simulink model "wlan_phy_rx_pmd"  (Simulink 7.8)
settings: Solver=VariableStepDiscrete  SolverMode=SingleTasking  StartTime=0.0  StopTime=simtime  RelTol=1e-3
PostLoadFcn:
  | wlan_phy_rx_init
InitFcn:
  | wlan_phy_rx_init
StartFcn:
  | wlan_phy_rx_init
values from neighbouring files (symbols this model uses):
  barker_seq20 = 0.95 * circshift(barker_seq20, [0 4]) ./ max(abs(barker_seq20))    [wlan_phy_rx_init.m]
  bit_scrambler_lfsr_bytes = bi2de(reshape(repmat(scr, 1, 8), 8, 127)', 'left-msb')    [wlan_phy_rx_init.m]
  deinterleave_ROM = [deinterleave_ROM interleave_64QAM zeros(1, 512-length(interleave_64QAM))]    [wlan_phy_rx_init.m]
  longCorr_coef_i = [3*real(LTS_corr)]    [wlan_phy_rx_init.m]
  longCorr_coef_q = [3*imag(LTS_corr)]    [wlan_phy_rx_init.m]
  MAX_CP_LEN = 32    [wlan_phy_rx_init.m]
  MAX_NCBPS = 288    [wlan_phy_rx_init.m]
  MAX_NUM_BYTES = 4096    [wlan_phy_rx_init.m]
  MAX_NUM_SC = 64    [wlan_phy_rx_init.m]
  MAX_NUM_SYMS = 600    [wlan_phy_rx_init.m]
  n_bits_preFFT_sampBuff = ceil(log2(4*MAX_NUM_SC))    [wlan_phy_rx_init.m]
  NCBPS_16QAM = 192    [wlan_phy_rx_init.m]
  NCBPS_64QAM = 288    [wlan_phy_rx_init.m]
  NCBPS_BPSK = 48    [wlan_phy_rx_init.m]
  NCBPS_QPSK = 96    [wlan_phy_rx_init.m]
  NDBPS_16QAM12 = 96    [wlan_phy_rx_init.m]
  NDBPS_16QAM34 = 144    [wlan_phy_rx_init.m]
  NDBPS_64QAM23 = 192    [wlan_phy_rx_init.m]
  NDBPS_64QAM34 = 216    [wlan_phy_rx_init.m]
  NDBPS_BPSK12 = 24    [wlan_phy_rx_init.m]
  NDBPS_BPSK34 = 36    [wlan_phy_rx_init.m]
  NDBPS_QPSK12 = 48    [wlan_phy_rx_init.m]
  NDBPS_QPSK34 = 72    [wlan_phy_rx_init.m]
  paylod_vec_samp_time = 8    [wlan_phy_rx_init.m]
  PHY_MIN_PKT_LEN = 14    [wlan_phy_rx_init.m]
  scr_ind_rev = zeros(1,128)    [wlan_phy_rx_init.m]
undefined symbols (not defined in the file or in its folder): gcbh, xlBlockLoadCallback, xlGetNormalizedPeriod, xlipgetpartsetting
libraries: xbsIndex_r4 x3519, simulink x1, xbsEDKLib_r4 x1
(part 1 of 21)
settings Model: Creator=murphpo  LastModifiedBy=murphpo
settings OptimizationCC: InlineParams=on
settings DebuggingCC: UnconnectedOutputMsg=none

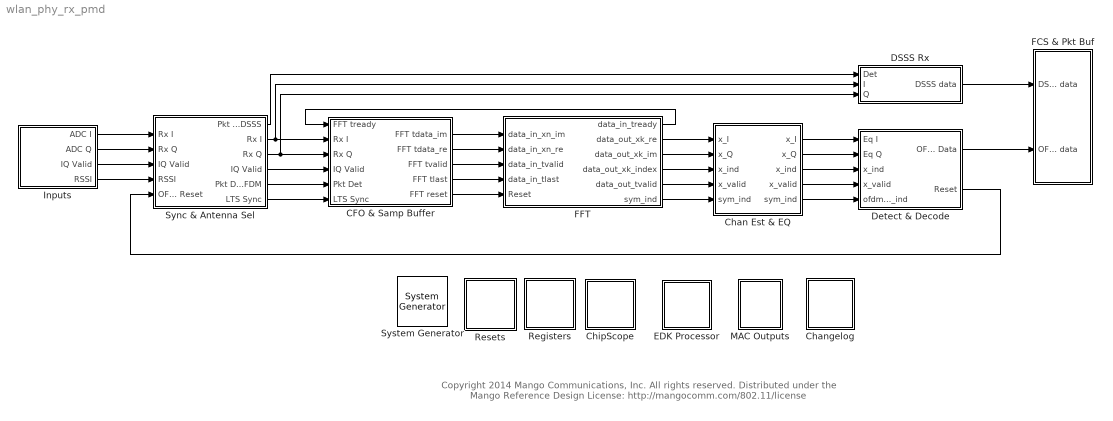
== system wlan_phy_rx_pmd ==
  note: Copyright 2014 Mango Communications, Inc. All rights reserved.
      |
      | Distributed under the Mango Reference Design License:
      | http://mangocomm.com/802.11/license
  " System Generator" : xbsIndex_r4/ System Generator  Tag=genX  xilinxfamily=virtex6  part=xc6vlx240t  speed=-2  package=ff1156  synthesis_tool=XST  clock_wrapper=Clock Enables  directory=./pcore_rx_v282  proj_type=Project Navigator  Synth_file=XST Defaults  Impl_file=ISE Defaults  testbench=off  simulink_period=1  sysclk_period=6.25  dcm_input_clock_period=10  incr_netlist=off  trim_vbits=Everywhere in SubSystem  dbl_ovrd=According to Block Masks  core_generation=According to Block Masks  run_coregen=off  deprecated_control=off  eval_field=0  block_type=sysgen
  CFO & Samp Buffer : Subsystem
  Chan Est & EQ : Subsystem
  Changelog : Subsystem
  ChipScope : Subsystem
  DSSS Rx : Subsystem
  Detect & Decode : Subsystem
  EDK Processor : Subsystem  LoadFcn=xlBlockLoadCallback(gcbh);  mask(Xilinx EDK Processor Block){mode=EDK pcore generation, xmp=, MemVisToProc=<qt bgcolor="#FFFFFF"><div><img src="S:/Xilinx/14.4/ISE_DS/ISE/sysgen/data/images/registerplus.gif"> &lt;&lt;DEBUG_GPIO&gt;&gt;<br></div><div><img src="S:/Xilinx/14.4/ISE_DS/ISE/sysgen/data/images/registerplus.gif"> &lt;&lt;RSSI_THRESH&gt;&gt;<br></div><div><img src="S:/Xilinx/14.4/ISE_DS/ISE/sysgen/data/images/registerplus.gif"> &lt;&lt;CONFIG&gt;&gt;<br></div><div><img src="S:/Xilinx/14.4/ISE_DS/ISE/sysgen/data/images/registerplus.gif"> &lt;&lt;DSSS_RX_CONFIG&gt;&gt;<br></div><div><img src="S:/Xilinx/14.4/ISE_DS/ISE/sysgen/data/images/registerplus.gif"> &lt;&lt;PKT_BUF_SEL&gt;&gt;<br></div><div><img src="S:/Xilinx/14.4/ISE_DS/ISE/sysgen/data/images/registerplus.gif"> &lt;&lt;PKTDET_AUTOCORR_CONFIG&gt;&gt;<br></div><div><img src="S:/Xilinx/14.4/ISE_DS/ISE/sysgen/data/images/registerplus.gif"> &lt;&lt;FEC_CONFIG&gt;&gt;<br></div><div><img src="S:/Xilinx/14.4/ISE_DS/ISE/sysgen/data/images/registerplus.gif"> &lt;&lt;Control&gt;&gt;<br></div><div><img src="S:/Xilinx/14.4/ISE_DS/ISE/sysgen/data/images/registerplus.gif"> &lt;&lt;LTS_Corr_Thresh&gt;&gt;<br></div><div><img src="S:/Xilinx/14.4/ISE_DS/ISE/sysgen/data/images/registerplus.gif"> &lt;&lt;PKTDET_DSSS_CONFIG&gt;&gt;<br></div><div><img src="S:/Xilinx/14.4/ISE_DS/ISE/sysgen/data/images/registerplus.gif"> &lt;&lt;LTS_Corr_Config&gt;&gt;<br></div><div><img src="S:/Xilinx/14.4/ISE_DS/ISE/sysgen/data/images/registerplus.gif"> &lt;&lt;PHY_CCA_CONFIG&gt;&gt;<br></div><div><img src="S:/Xilinx/14.4/ISE_DS/ISE/sysgen/data/images/registerplus.gif"> &lt;&lt;FFT_Config&gt;&gt;<br></div><div><img src="S:/Xilinx/14.4/ISE_DS/ISE/sysgen/data/images/registerplus.gif"> &lt;&lt;PKTDET_RSSI_CONFIG&gt;&gt;<br></div><div><img src="S:/Xilinx/14.4/ISE_DS/ISE/sysgen/data/images/registerplus.gif"> &lt;&lt;RX_PKT_RSSI_CD&gt;&gt;<br></div><div><img src="S:/Xilinx/14.4/ISE_DS/ISE/sysgen/data/images/registerplus.gif"> &lt;&lt;RX_PKT_AGC_GAINS&gt;&gt;<br></div><div><img src="S:/Xilinx/14.4/ISE_DS/ISE/sysgen/data/images/registerplus.gif"> &lt;&lt;RX_PKT_RSSI_AB&gt;&gt;<br></div><div><img src="S:/Xilinx/14.4/ISE_DS/ISE/sysgen/data/images/registerplus.gif"> &lt;&lt;Status&gt;&gt;<br></div></qt>, AvailableMemories=<empty>, portInterfaceTable={'exposed'=>[],'portdir'=>[],'portname'=>[],'shortname'=>[]}, bus_type_sgadvanced=, bus_type=AXI, baseaddr=0x80000000, baseaddr_lock_sgadvanced=, baseaddr_lock=off, dual_clock_sgadvanced=, dual_clock=on, reg_readback_sgadvanced=, reg_readback=on, ucf_file=, inheritDeviceType_sgadvanced=, inheritDeviceType=off, elf_file=, codebug_sgadvanced=, codebug=on, clock_name=plb, internalPortList={}, resetPolarity=0, memxtable={'mladdr'=>[0.00000000000000000,1.00000000000000000,2.00000000000000000,3.00000000000000000,4.00000000000000000,5.00000000000000000,6.00000000000000000,7.00000000000000000,8.00000000000000000,9.00000000000000000,10.00000000000000000,11.00000000000000000,12.00000000000000000,13.00000000000000000,0.00000000000000000,1.00000000000000000,2.00000000000000000,3.00000000000000000],'mlist'=>['wlan_phy_rx_pmd/Registers/From Register9','wlan_phy_rx_pmd/Registers/From Register8','wlan_phy_rx_pmd/Registers/From Register7','wlan_phy_rx_pmd/Registers/From Register6','wlan_phy_rx_pmd/Registers/From Register5','wlan_phy_rx_pmd/Registers/From Register4','wlan_phy_rx_pmd/Registers/From Register3','wlan_phy_rx_pmd/Registers/From Register2','wlan_phy_rx_pmd/Registers/From Register14','wlan_phy_rx_pmd/Registers/From Register13','wlan_phy_rx_pmd/Registers/From Register12','wlan_phy_rx_pmd/Registers/From Register11','wlan_phy_rx_pmd/Registers/From Register1','wlan_phy_rx_pmd/Registers/From Register','wlan_phy_rx_pmd/Registers/To Register4','wlan_phy_rx_pmd/Registers/To Register3','wlan_phy_rx_pmd/Registers/To Register2','wlan_phy_rx_pmd/Registers/To Register'],'mlname'=>['\\'DEBUG_GPIO\\'','\\'RSSI_THRESH\\'','\\'CONFIG\\'','\\'DSSS_RX_CONFIG\\'','\\'PKT_BUF_SEL\\'','\\'PKTDET_AUTOCORR_CONFIG\\'','\\'FEC_CONFIG\\'','\\'Control\\'','\\'LTS_Corr_Thresh\\'','\\'PKTDET_DSSS_CONFIG\\'','\\'LTS_Corr_Config\\'','\\'PHY_CCA_CONFIG\\'','\\'FFT_Config\\'','\\'PKTDET_RSSI_CONFIG\\'','\\'RX_PKT_RSSI_CD\\'','\\'RX_PKT_AGC_GAINS\\'','\\'RX_PKT_RSSI_AB\\'','\\'Status\\''],'mlstate'=>[0.00000000000000000,0.00000000000000000,0.00000000000000000,0.00000000000000000,0.00000000000000000,0.00000000000000000,0.00000000000000000,0.00000000000000000,0.00000000000000000,0.00000000000000000,0.00000000000000000,0.00000000000000000,0.00000000000000000,0.00000000000000000,0.00000000000000000,0.00000000000000000,0.00000000000000000,0.00000000000000000]}, procinfo={}, memmapdirty=off, blockname=, xpsintstyle=default, has_advanced_control=0, sggui_pos=-1,-1,-1,-1, block_type=edkprocessor, block_version=2.7, sg_icon_stat=45,45,-1,-1,white,blue,0,07734,right,,[ ],[ ], sg_mask_display=|, sg_list_contents={'table'=>{'AvailableMemories'=>'popup(<empty>)'}}, sg_blockgui_xml=}
      mask sg_mask_display=|
      | fprintf('','COMMENT: begin icon graphics');
      | patch([0 45 45 0 0 ],[0 0 45 45 0 ],[0.77 0.82 0.91 ]);
      | plot([0 45 45 0 0 ],[0 0 45 45 0 ]);
      | patch([8.65 17.32 23.32 29.32 35.32 23.32 14.65 8.65 ],[28.66 28.66 34.66 28.66 34.66 34.66 34.66 28.66 ],[1 1 1 ]);
      | patch([14.65 23.32 17.32 8.65 14.65 ],[22.66 22.66 28.66 28.66 22.66 ],[0.931 0.946 0.973 ]);
      | patch([8.65 17.32 23.32 14.65 8.65 ],[16.66 16.66 22.66 22.66 16.66 ],[1 1 1 ]);
      | patch([14.65 35.32 29.32 23.32 17.32 8.65 14.65 ],[10.66 10.66 16.66 10.66 16.66 16.66 10.66 ],[0.931 0.946 0.973 ]);
      | fprintf('','COMMENT: end icon graphics');
      | fprintf('','COMMENT: begin icon text');
      | fprintf('','COMMENT: end icon text');
      mask prompts: mode "Configure Processor for"; xmp "XPS Project"; MemVisToProc ""; AvailableMemories "Available Memories"; portInterfaceTable ""; bus_type_sgadvanced ""; bus_type "Bus Type"; baseaddr "Base Address"; baseaddr_lock_sgadvanced ""; baseaddr_lock "Lock"; dual_clock_sgadvanced ""; dual_clock "Dual Clocks"; reg_readback_sgadvanced ""; reg_readback "Register Read-Back"; ucf_file "Constraint File"; inheritDeviceType_sgadvanced ""; inheritDeviceType "Inherit Device Type"; elf_file "Initial Program"; codebug_sgadvanced ""; codebug "Enable Co-Debug with Xilinx SDK (Beta)"; clock_name ""; internalPortList ""; resetPolarity ""; memxtable ""; procinfo ""; memmapdirty ""; blockname ""; xpsintstyle ""; has_advanced_control ""; sggui_pos ""; block_type ""; block_version ""; sg_icon_stat ""; sg_mask_display ""; sg_list_contents ""; sg_blockgui_xml ""
      mask help: Xilinx EDK Processor
      mask init:
      | try
      |   tmp_gcb = gcb;
      |   tmp_gcbh = gcbh;
      |   if (strcmp('SysGenIndex',get_param(bdroot(tmp_gcbh),'tag')) && ~isempty(regexp(bdroot(tmp_gcb), '^xbs', 'once')))
      |     return;
      |   end;
      |   xlMungeMaskParams;
      | 
      |   block_type='edkprocessor';
      | 
      |   serialized_declarations = '{''block_type''=>''String''}';
      |   xledkprocessor_init();
      |   ptable_ = xlblockprep(get_param(tmp_gcb, 'MaskWSVariables'));
      |   try
      |     xlBlockMoveCallback(tmp_gcbh);
      |   catch 
      |      clear global xl_updateicon_recursion_guard;
      |   end;
      | catch
      |   global dbgsysgen;
      |   if(~isempty(dbgsysgen) && dbgsysgen)
      |     e = regexprep(lasterr, '\n', '\nError: ');
      |     disp(['Error: While running MaskInit code on block ' tmp_gcb ': ' e]);
      |     error(e);
      |   end
      | end
      settings: CopyFcn=xlProcBlockCopyCallback(gcbh);xlBlockMoveCallback(gcbh);  DeleteFcn=xlDestroyGui(gcbh);  ModelCloseFcn=xlDestroyGui(gcbh);  PreSaveFcn=xlBlockPreSaveCallback(gcbh);  PostSaveFcn=xlBlockPostSaveCallback(gcbh);  DestroyFcn=xlDestroyGui(gcbh);  OpenFcn=bh=gcbh;xlProcBlockCallbacks('populatesharedmemorylistbox',bh);xlOpenGui(bh, 'edkprocessor_gui.xml', @xlProcBlockEnablement, @xlProcBlockAction);  CloseFcn=xlDestroyGui(gcbh);  MoveFcn=xlBlockMoveCallback(gcbh);
      mask Help: eval('');xlDoc('-book','sysgen','-topic','EDK_Processor');
      mask StyleString: popup(EDK pcore generation|HDL netlisting),edit,edit,edit,edit,edit,popup(AXI|PLB),edit,edit,checkbox,edit,checkbox,edit,checkbox,edit,edit,checkbox,edit,edit,checkbox,edit,edit,edit,edit,edit,edit,edit,edit,edit,edit,edit,edit,edit,edit,edit,edit
      mask Display:
      | fprintf('','COMMENT: begin icon graphics');
      | patch([0 45 45 0 0 ],[0 0 45 45 0 ],[0.77 0.82 0.91 ]);
      | plot([0 45 45 0 0 ],[0 0 45 45 0 ]);
      | patch([8.65 17.32 23.32 29.32 35.32 23.32 14.65 8.65 ],[28.66 28.66 34.66 28.66 34.66 34.66 34.66 28.66 ],[1 1 1 ]);
      | patch([14.65 23.32 17.32 8.65 14.65 ],[22.66 22.66 28.66 28.66 22.66 ],[0.931 0.946 0.973 ]);
      | patch([8.65 17.32 23.32 14.65 8.65 ],[16.66 16.66 22.66 22.66 16.66 ],[1 1 1 ]);
      | patch([14.65 35.32 29.32 23.32 17.32 8.65 14.65 ],[10.66 10.66 16.66 10.66 16.66 16.66 10.66 ],[0.931 0.946 0.973 ]);
      | fprintf('','COMMENT: end icon graphics');
      | fprintf('','COMMENT: begin icon text');
      | disp('');
      | 
      | fprintf('','COMMENT: end icon text');
  FCS & Pkt Buf : Subsystem
  FFT : Subsystem
  Inputs : Subsystem
  MAC Outputs : Subsystem
  Registers : Subsystem
  Resets : Subsystem
  Sync & Antenna Sel : Subsystem
  wires:
  FFT/1(data_in_tready) -> CFO & Samp Buffer/1(FFT tready)
  CFO & Samp Buffer/4(FFT tlast) -> FFT/4(data_in_tlast)
  CFO & Samp Buffer/1(FFT tdata_im) -> FFT/1(data_in_xn_im)
  CFO & Samp Buffer/2(FFT tdata_re) -> FFT/2(data_in_xn_re)
  CFO & Samp Buffer/3(FFT tvalid) -> FFT/3(data_in_tvalid)
  Chan Est & EQ/1(" x_I") -> Detect & Decode/1(Eq I)
  Chan Est & EQ/2(" x_Q") -> Detect & Decode/2(Eq Q)
  Chan Est & EQ/3(" x_ind") -> Detect & Decode/3(x_ind)
  Chan Est & EQ/4(" x_valid") -> Detect & Decode/4(x_valid)
  Chan Est & EQ/5(" sym_ind") -> Detect & Decode/5(ofdm_sym_ind)
  FFT/2(data_out_xk_re) -> Chan Est & EQ/1(x_I)
  FFT/3(data_out_xk_im) -> Chan Est & EQ/2(x_Q)
  FFT/4(data_out_xk_index) -> Chan Est & EQ/3(x_ind)
  FFT/5(data_out_tvalid) -> Chan Est & EQ/4(x_valid)
  FFT/6(sym_ind) -> Chan Est & EQ/5(sym_ind)
  DSSS Rx/1(DSSS data) -> FCS & Pkt Buf/1(DSSS data)
  Detect & Decode/1(OFDM Data) -> FCS & Pkt Buf/2(OFDM data)
  Detect & Decode/2(" Reset") -> Sync & Antenna Sel/5(OFDM Reset)
  Inputs/1(ADC I) -> Sync & Antenna Sel/1(Rx I)
  Inputs/2(ADC Q) -> Sync & Antenna Sel/2(Rx Q)
  Inputs/3(IQ Valid) -> Sync & Antenna Sel/3(IQ Valid)
  Inputs/4(RSSI) -> Sync & Antenna Sel/4(RSSI)
  CFO & Samp Buffer/5(FFT reset) -> FFT/5(Reset)
  Sync & Antenna Sel/2(" Rx I") -> CFO & Samp Buffer/2(Rx I), DSSS Rx/2(I)
  Sync & Antenna Sel/3(" Rx Q") -> CFO & Samp Buffer/3(Rx Q), DSSS Rx/3(Q)
  Sync & Antenna Sel/4(" IQ Valid") -> CFO & Samp Buffer/4(IQ Valid)
  Sync & Antenna Sel/5(" Pkt Det OFDM") -> CFO & Samp Buffer/5(Pkt Det)
  Sync & Antenna Sel/6(LTS Sync) -> CFO & Samp Buffer/6(LTS Sync)
  Sync & Antenna Sel/1(" Pkt Det DSSS") -> DSSS Rx/1(Det)

== system wlan_phy_rx_pmd/CFO & Samp Buffer ==
  note: FIXME:
      | -DDS runs 1 extra cycle during LTS; it's right for post-LTS FFT inputs
  FFT tready : Inport
  Rx I : Inport  Port=2
  Rx Q : Inport  Port=3
  IQ Valid : Inport  Port=4
  Pkt Det : Inport  Port=5
  LTS Sync : Inport  Port=6
  ADC/Corr : Scope  NumInputPorts=8
      settings: TimeRange=10000  YMin=-1~-1~0~-1~-1~-1~-1~-1  YMax=1~1~1~1~1~1~1~1  SaveName=ScopeData8
  CFO Correction : Subsystem
  CFO Estimation : Subsystem
  FFT in FIFO Ctrl : Scope  NumInputPorts=7
      settings: TimeRange=30000  YMin=0~0~0~-0.1~0~-0.1~-0.1  YMax=300~300~1~1.1~4000~1.1~1.1
  FIFO : Subsystem
  Gateway Out{1..5,7,9..11,17} (10 alike) : xbsIndex_r4/Gateway Out  inherit_from_input=off  hdl_port=off  timing_constraint=None  locs_specified=off  LOCs={}  UseAsDAC=off  DACChannel='1'  block_type=gatewayout
  Inverter : xbsIndex_r4/Inverter  en=off  latency=0  dbl_ovrd=off  block_type=inv
  Logical2 : xbsIndex_r4/Logical  logical_function=OR  inputs=2  en=off  latency=0  precision=Full  arith_type=Unsigned  n_bits=16  bin_pt=0  align_bp=on  dbl_ovrd=off  block_type=logical
  Negedge : Subsystem
  Samp Buffer : Subsystem
  Slice1 : xbsIndex_r4/Slice  nbits=1  boolean_output=on  mode=Lower Bit Location + Width  bit1=0  base1=MSB of Input  bit0=0  base0=LSB of Input  dbl_ovrd=off  block_type=slice
  Slice2 : xbsIndex_r4/Slice  nbits=1  boolean_output=on  mode=Upper Bit Location + Width  bit1=0  base1=MSB of Input  bit0=0  base0=LSB of Input  dbl_ovrd=off  block_type=slice
  FFT tdata_im : Outport
  FFT tdata_re : Outport  Port=2
  FFT tvalid : Outport  Port=3
  FFT tlast : Outport  Port=4
  FFT reset : Outport  Port=5
  wires:
  Rx Q/1 -> CFO Estimation/3(rx_Q), Samp Buffer/4(rx Q), Gateway Out7/1
  Rx I/1 -> Samp Buffer/3(rx I), CFO Estimation/2(rx_I), Gateway Out17/1
  FFT tready/1 -> FIFO/5(FFT tready), Gateway Out9/1
  Samp Buffer/1(I) -> CFO Correction/1("I ")
  Samp Buffer/2(Q) -> CFO Correction/2("Q ")
  CFO Correction/2(I) -> FIFO/2(I)
  CFO Correction/3(Q) -> FIFO/3(Q)
  CFO Correction/1(valid) -> FIFO/1(data tvalid), Gateway Out4/1
  Samp Buffer/5(tlast) -> CFO Correction/5(tlast)
  Samp Buffer/4(" IQ valid") -> CFO Correction/4(Valid)
  FIFO/5(FIFO tready) -> Samp Buffer/7(FIFO tready)
  FIFO/4(FFT tlast) -> FFT tlast/1, Gateway Out2/1
  FIFO/3(FFT tdata_im) -> FFT tdata_im/1
  FIFO/1(FFT tvalid) -> FFT tvalid/1, Gateway Out1/1
  FIFO/2(FFT tdata_re) -> FFT tdata_re/1
  Gateway Out3/1 -> FFT in FIFO Ctrl/1  "FIFO IN: tlast"
  Gateway Out4/1 -> FFT in FIFO Ctrl/2  "FIFO IN: valid"
  IQ Valid/1 -> CFO Estimation/4(rx_valid), Samp Buffer/5(IQ valid), Gateway Out5/1
  Slice1/1 -> CFO Estimation/1(LTS_Sync)
  Slice2/1 -> Samp Buffer/6(LTS Sync 2)
  Gateway Out17/1 -> ADC/Corr/1  "ADC I"
  Gateway Out7/1 -> ADC/Corr/2  "ADC Q"
  Gateway Out5/1 -> ADC/Corr/3  "ADC Valid"
  Samp Buffer/3(Buff Rd) -> CFO Correction/3(DDS Run)
  CFO Correction/4(" tlast") -> FIFO/4(data tlast), Gateway Out3/1
  Gateway Out1/1 -> FFT in FIFO Ctrl/3  "FIFO Out: valid"
  Gateway Out2/1 -> FFT in FIFO Ctrl/4  "FIFO Out: tlast"
  Gateway Out9/1 -> FFT in FIFO Ctrl/5  "FFT in: tready"
  Logical2/1 -> Gateway Out11/1, Samp Buffer/2(Pkt Done)
  Gateway Out10/1 -> ADC/Corr/4  "Pkt Det"
  Gateway Out11/1 -> ADC/Corr/7  "Pkt done"
  Inverter/1 -> FIFO/6(reset), FFT reset/1
  [RX_GLOBAL_RESET] -> Logical2/1
  Negedge/1(Q) -> Logical2/2
  CFO Estimation/1(CFO) -> CFO Correction/6(CFO Est)
  LTS Sync/1 -> Slice2/1, Slice1/1
  Pkt Det/1 -> Samp Buffer/1(Pkt Det), Negedge/1(D), Gateway Out10/1, Inverter/1

== system wlan_phy_rx_pmd/Chan Est & EQ ==
  x_I : Inport
  x_Q : Inport  Port=2
  x_ind : Inport  Port=3
  x_valid : Inport  Port=4
  sym_ind : Inport  Port=5
  Chan Est : Subsystem
  EQ & Pilot Processing : Subsystem
  " x_I" : Outport
  " x_Q" : Outport  Port=2
  " x_ind" : Outport  Port=3
  " x_valid" : Outport  Port=4
  " sym_ind" : Outport  Port=5
  wires:
  EQ & Pilot Processing/1(" x_I") -> " x_I"/1
  EQ & Pilot Processing/2(" x_Q") -> " x_Q"/1
  Chan Est/3(H_I) -> EQ & Pilot Processing/3(H_I)
  Chan Est/4(H_Q) -> EQ & Pilot Processing/4(H_Q)
  Chan Est/5(" valid") -> EQ & Pilot Processing/5(x_valid)
  Chan Est/6(" x_ind") -> EQ & Pilot Processing/6(x_ind)
  Chan Est/7(" sym_ind") -> EQ & Pilot Processing/7(Sym ind)
  Chan Est/1(" x_I") -> EQ & Pilot Processing/1(x_I)
  Chan Est/2(" x_Q") -> EQ & Pilot Processing/2(x_Q)
  x_I/1 -> Chan Est/1(x_re)
  x_Q/1 -> Chan Est/2(x_im)
  x_ind/1 -> Chan Est/3(x_ind)
  x_valid/1 -> Chan Est/4(x_valid)
  sym_ind/1 -> Chan Est/5(Sym Ind)
  EQ & Pilot Processing/3(" x_ind") -> " x_ind"/1
  EQ & Pilot Processing/4(" x_valid") -> " x_valid"/1
  EQ & Pilot Processing/5(" sym ind") -> " sym_ind"/1

== system wlan_phy_rx_pmd/Changelog ==
  note: 2.00.b:
      | Same as 2.00.a but with ILA removed (for MAC debug)
      |
      | 2.00.c:
      | Same as 2.00.b but with ILA re-enabled
      |
      | 2.00.d:
      | -Reworked auto-corr pkt det data types to avoid overflow and allow thresholds
      |   to cover full range of mag/corr values observed in hw
      |
      | 2.00.e:
      | Same as 2.00.d but with ILA removed
      |
      | 2.00.f:
      | -Re-designed CFO est to take phase of average of conj mults (should be better
      |   performance at low SNR)
      |
      | 2.00.g:
      | -Added CFG bit to bypass CFO est
      | -Re-enabled ILA
      |
      | 2.00.h:
      | -Fixed zero_tail and num_bytes bug that flagged 2/3 of pkt lens bad
      |
      | 2.00.i:
      | -Fixed rx_end timing bug that asserted end before FCS for some lengths
      | -Redesigned DSSS and OFDM pkt buf and pkt done logic
      | -Added DSSS bytes to FCS check
      | -Removed pkt done state - now handled by MAC
      | -Added DSSS length to SIGNAL register
      |
      | 2.00.j:
      | -Removed SIGNAL register (now handed by MAC)
      | -Fixed DSSS/FCS bug (VIN was high 8 cycles per byte)
      |
      | 2.00.k:
      | -Fixed rx_start rate/length output timing for OFDM rx
      | -Bypassed OFDM descrabler for SIGNAL field bytes (raw SIGNAL now
      |    written to pkt buf)
      |
      | 2.00.l:
      | -Added 64-bit pkt buf interface option (32b is disregarded subsystem in this export)
      | -Added pkt buf addr offset, common to all pkt bufs, so MAC can store per-pkt metadata
      |
      | 2.00.m:
      | -Re-designed all post-EQ logic (demod, de-interleave, decode) to support other mod schemes
      | -Re-defined FEC_Config register; now only stores scaling values
      |
      | 2.00.n:
      | -Added pipeline regs at decoder input to resolve timing errors
      |
      | 2.00.o:
      | -Re-enabled CRC checking in DSSS headers, extended max length of DSSS pkts (now 1500 bytes, not bits)
      |
      | 2.00.p:
      | -Removed protection DFF's between pkt buf sel regs and bram addr gen; it's now software's job to be safe
      | -Bit-order-swapped the RATE output to MAC
      |
      | 2.00.q:
      | -Fixed interpretation of RATE values in m-code block to match new bit-un-reversed values
      |
      | 2.00.r:
      | -Fixed SIGNAL init values (wasn't a problem, just inconsistent)
      | -Robustified byte counter and byte_valid at decoder to stop after num bytes, even if decoder outputs extra bytes
      | -Added OFDM_Running -> DSSS_Reset control
      |
      | 2.00.s:
      | -chunter export
      |
      | 2.00.t:
      | -Nuked Sysgen caches, re-exported
      |
      | 2.00.u:
      | -Bypassed de-scrambler for SIGNAL bytes (now SIGNAL is written to pkt buf for use in software)
      |
      | 2.00.v:
      | -Tweaked reset logic for de-interleave addr gen (still chasing problem of corruption in Rx bytes at end
      |   of pkts > 105 bytes at Q34 in hardware, but not in sim...)
      |
      | 2.00.w:
      | -Moved CS.RSSI to  post RSSI sum
      | -Registered LEN/RATE on valid SIGNAL, reset to sane defaults every pkt
      | -Robustified fake Rx busy logic (can't start outside pkt det, stops running when done)
      |
      | 2.00.x:
      | -Fixed bug in SIGNAL invalid checking
      | -Added SIGNAL value to register
      |
      | 2.00.y:
      | -Added more FFT/decoder ctrl signals to ChipScope
      |
      | 2.00.z:
      | -Added even more demod/deint signals to CS ILA
  note: 2.02.a:
      | -Disabled aresetn port on de-int sym FIFO
      |
      | 2.02.b:
      | -More CS signals
      |
      | 2.02.c:
      | -Yet more CS signals
      |
      | 2.02.d:
      | -Reworked RSSI det reset logic (posedge -> SR latch, simpler reset)
      | -Reworked DSSS reset logic (now has 128 cycle reset pulse every time)
      | -Reworked DSSS RX_END indication; every DSSS RX_START will lead to
      |   RX_END, even intra-Rx reset events (FCS will be bad, but RX_ED will occur)
      |
      | 2.02.e:
      | -Teweaked rx_ended_unexpectedly logic to require pkt_det = 1, to avoid sending
      |   rx_end.error if MAC blocks Rx post-good-fcs, pre-last-extended-samp
      | -Added MAC rx_start/fcs/end to CS ILA
      |
      | 2.02.f:
      | Re-export, no modifications; trying to debug per-build bad states
      |
      | 2.02.g:
      | -Re-added RSSI to CS ILA to debug pkt det issues
      |
      | 2.02.h:
      | -Changed pkt det reset extension to be negedge(pkt det reset), so every reset event
      |   (whether 1 or thousands of cycles) gets extended from its end, not its beginning
      | -Routed debug[0] to CS ILA trigger port, so sw can trigger CS
      |
      | 2.02.i:
      | -Added Rx_Sigs_Invalid input from Tx PHY to block ADC/RSSI inputs during TxEn
      |   (only honored when USE_ bit is set in config register)
      | -Added LTS and Rx_Sigs_Invalid sigs to CS ILA
      |
      | 2.02.j:
      | -Reworked pre-FFT samp buf control to handle cases of late pkt det (this was the
      |   cause of Rx deadlock, when post-Tx Rx events happened too soon)
      |
      | 2.02.k:
      | -Added Rx ctrl sigs back to ILA, to debug occasional long pkt det events in hardware
      |   (they look like perma-dets, but the Rx PHY somehow recovers)
      |
      | 2.02.l:
      | -Made decoder nrst synchronous throughout (chasing possible bug in SIGNAL parity
      |   error before last SIGNAL byte is written...it's a long shot)
      |
      | 2.02.m:
      | -Added 16-QAM support
      |
      | 2.02.n:
      | -Added more robust FFT reset logic to handle case of mid-FFT RX_END event
      |
      | 2.02.o:
      | -Redesigned pilot phase error est and correction (pilots now correct same symbol)
      | -Fixed bugs in LTS correlator (lower thresholds!)
      | -Added high/SNR thresholds for LTS corr (new register!)
      | -Merged pilot phase error calc and EQ to avoid having two conj mults
      | -Added dbg_SIGNAL_ERR_DISP gw out
      |
      | 2.02.p:
      | -Disabled aresetn on pre-FFT sample FIFO (trying to resolve sim/hw mismatch for
      |   16Q Rx errors with certain payload lengths)
      |
      | 2.02.q:
      | -Added DFF in phase error calc to resolve timing issues
      |
      | 2.02.r:
      | -Added more latency in phase error accumulators for timing (2.00.p and q missed timing
      |   by 6+ nsec)
      | -Switched FP add/subs to use DSP48
      |
      | 2.02.s, t:
      | -Yet more latency in FP cores
      |
      | 2.02.u:
      | -Redesigned deinterleaver for lower latency
      | -Added 64QAM support
      |
      | 2.02.v,w,x:
      | -Updated CS sigs to debug always-bad-SIGNAL in hw
      |
      | 2.02.y:
      | -Registered output of SIGNAL rate decode, trying to fix unsimulatable behavior in sym FIFO
      |
      | 2.02.z:
      | -Tweaked BPSK demap to resolve (I hope) hw/sim mismatch
      |
      | 2.03.a:
      | -Increased sym fifo depth to 128 (grasping here...)
      |
      | 2.03.b,c:
      | -Added more de-int sigs to ILA
      |
      | 2.03.d:
      | -Added explicit reset to demappers - should help resolve all-bad-after-one-bad behavior in hw
  note: 2.03.e:
      | -Re-designed de-interleaver control to fix rate=2/3 bug
      |
      | 2.03.f:
      | -Modified decoder blackbox
      |   -Changed default traceback to 48 (K*7 - 1)
      |   -Added early_trace ctrl signal to start early 24 bit traceback for SIGNAL
      | -Redesigned decoder control to stop vin after last symbol and trigger pkt_end
      |   (reduces latency to last input -> last output, required to meet SIFS at 64Q)
      |
      | 2.03.g:
      | -Added logic to store chan estimates per Rx event
      | -Removed unused 32-bit pktBuf interface
      |
      | 2.03.h:
      | -Updated H est offset to be byte value (matching rx_header offset)
      | -Added bit to en/disable u32 order swap for H Est writing
      | -Added selection diversity
      |   -Auto-select Rx ant based on AGC gains
      |   -Dual pkt det subsystems
      | -Split registers for post-AGC gains and RSSI, to capture RF A and B
      | -Added FIFO to buffer/downsample EQ I/Q debug outputs for 40MS DAC outputs
      |
      | 2.03.i:
      | -Added RF B RSSI to CCA
      |
      | 2.03.j:
      | -Fixed bit sel bug in Rx CFG reg for RF B pkt det En
      |
      | 2.03.k:
      | -Fixed AGC gain capture bug (was always capturing 0, one cycle early)
      |
      | 2.03.l:
      | -Fixed FFT reset error for certain rate/length combos
      |
      | 2.03.m:
      | -Added ext pkt det signal
      | -Cleaned up CFO est block
      | -Removed unused reset port
      |
      | 2.03.n:
      | -Updated auto-corr pkt det energy thresh datatype to UFix14_8 (from UFix12_0)
      | -Reduced aut-corr pkt min dur type to UFix4_0 (from UFix6_0; value is 4 in ref des)
      | -Updated LTS correlator to support arbitrary sample rates (still requires samp_rate<=(sys_clk/8))
      |
      | 2.03.o:
      | -Fixed descrambler reset bug, that caused wrong bad FCS events (at worst) every 1/126 pkts
      |
      | 2.03.p:
      | -Added RFC/RFD inputs
      | -Added ext ADC_RX_CLK port to control sampling rate
      | -Moved ext pkt det enable bit to config[13]
      | -Added PHY CCA mode select (0=any enabled antenna, 1=all enabled antennas)
      | -Added pkt det status reg bits, to read per-Rx which antenna was selected
      | -Moved non-switching-ant-sel to config[16:15]
      | -Added PHY CCA busy calc mode (any vs all enabled antennas > threshold)
      | -Added DSSS active debug output
      | -Added post-AGC-RSSI_CD register (_AB was already there)
      | -Swapped some chipscope signals (added MAC rate, removed symfifo status)
      |
      | 2.03.q:
      | -Moved CS_ADC_x to pre-IQ-valid-qualified signals
      | -Fixed bit numbering bug for pktDet_En on RFC/D
      |
      | 2.03.r:
      | -More pipelineing in DSSS Rx for easier timing
      |
      | 2.03.s:
      | -Yet more pipeline registers, mostly at software accessible registers I/O
      |
      | 2.03.t:
      | -Explicit fanout regs for some sw regs for better timing
      |
      | 2.03.u
      | -More pipeline regs on top level outputs (mostly debug signals that could even be TIG)
      | -Debug copy of FCS_Good signal for header output w/ extra regs
      |
      | 2.03.v
      | -Major rework of pkt det - seperate autocorr logic/thresholds for OFDM vs DSSS
      | -DSSS Rx now requires DSSS pkt det before starting
      | -Updated registers for pkt det and DSSS Rx
      | -Separate debug outputs for OFDM and DSSS pkt det
      | -Made max SIGNAL length value programmable (in units of kB)
      | -Removed SIGNAL field register (wasn't used anymore - all Rx bytes are in pkt buf)
      |
      | 2.03.w:
      | -Updated DSSS autocorr threshold data types to handle high-SNR Rx
      | -Added DSSS reset for autocorr_det but no sym sync - resets after 4 bit intervals
      | -Tweaked chipscope signals for better visibility of OFDM vs DSSS Rx activity
      |
      | 2.03.x:
      | -Fixed DSSS reset blocking OFDM pkt det (separated min duration coutners for DSSS/OFDM)
      | -Fixed DSSS pkt det signalt to CS ILA
  note: 2.03.y:
      | -Extended DSSS byte_valid to 8 cycles (trying to debug apparent failure of some DSSS payloads
      |   to be writte to BRAM...)
      |
      | 2.03.z:
      | -Fixed dumb error in 2.03.y export (DSSS det was tied high for sim, broken in hw)
      |
      | 2.04.a:
      | -More itereating on DSSS data output logic
      | -Added min SIGNAL.LENGTH (14 bytes) to DSSS decoder
      |
      | 2.04.b:
      | -Added DFF to ext pkt det reset debounce logic (fixed timing failure)
      |
      | 2.04.c:
      | -Added DFFs for ext pkt det input (failed timing)

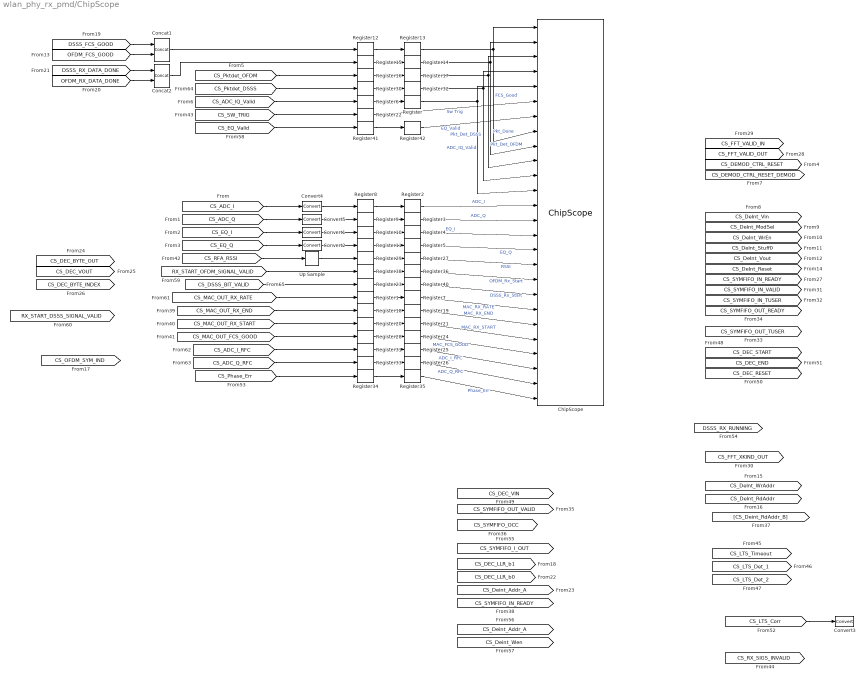
== system wlan_phy_rx_pmd/ChipScope ==
  ChipScope : xbsIndex_r4/ChipScope  num_trig_ports=7  current_port=3  show_trig_port=0  match_units=1  match_type=Basic with edges  data_is_trigger=off  num_data_ports=19  data_depth=16384  SRL16s=on  add_bufg=on  match_type_t0=2  match_type_t1=2  match_type_t2=2  match_type_t3=2  match_type_t4=2  match_type_t5=2  match_type_t6=1  match_type_t7=1  match_type_t8=1  match_type_t9=1  match_type_t10=1  match_type_t11=1  match_type_t12=1  match_type_t13=1  match_type_t14=1  match_type_t15=1  match_units_t0=1  match_units_t1=1  match_units_t2=1  match_units_t3=1  match_units_t4=1  match_units_t5=1  match_units_t6=1  match_units_t7=1  match_units_t8=1  match_units_t9=1  match_units_t10=1  match_units_t11=1  match_units_t12=1  match_units_t13=1  match_units_t14=1  match_units_t15=1  dbl_ovrd=0  user_scan_chain=USER1  block_type=chipscope
      settings: infoedit="Enables run-time debugging and verification of signals by inserting ChipScope Pro ICON and ILA cores.<br><br>  Restrictions: <br>Only one ChipScope core can be instantiated in a System Generator design. A design or subsystem containing a ChipScope block must have at least one output port."
  Concat1 : xbsIndex_r4/Concat  num_inputs=2  dbl_ovrd=off  block_type=concat
      settings: block_version=8.2.02
  Concat2 : xbsIndex_r4/Concat  num_inputs=2  dbl_ovrd=off  block_type=concat
      settings: block_version=8.2.02
  Convert1 : xbsIndex_r4/Convert  gui_display_data_type=Fixed-point  arith_type="Signed  (2's comp)"  n_bits=16  bin_pt=14  float_type=Single  exp_bits=8  fraction_bits=24  quantization=Truncate  overflow=Wrap  en=off  latency=0  dbl_ovrd=off  pipeline=off  block_type=convert
  Convert2 : xbsIndex_r4/Convert  gui_display_data_type=Fixed-point  arith_type="Signed  (2's comp)"  n_bits=16  bin_pt=14  float_type=Single  exp_bits=8  fraction_bits=24  quantization=Truncate  overflow=Wrap  en=off  latency=0  dbl_ovrd=off  pipeline=off  block_type=convert
  Convert3 : xbsIndex_r4/Convert  gui_display_data_type=Fixed-point  arith_type=Unsigned  n_bits=15  bin_pt=0  float_type=Single  exp_bits=8  fraction_bits=24  quantization=Truncate  overflow=Wrap  en=off  latency=0  dbl_ovrd=off  pipeline=off  block_type=convert
  Convert4 : xbsIndex_r4/Convert  gui_display_data_type=Fixed-point  arith_type="Signed  (2's comp)"  n_bits=12  bin_pt=11  float_type=Single  exp_bits=8  fraction_bits=24  quantization=Truncate  overflow=Wrap  en=off  latency=0  dbl_ovrd=off  pipeline=off  block_type=convert
  Convert5 : xbsIndex_r4/Convert  gui_display_data_type=Fixed-point  arith_type="Signed  (2's comp)"  n_bits=12  bin_pt=11  float_type=Single  exp_bits=8  fraction_bits=24  quantization=Truncate  overflow=Wrap  en=off  latency=0  dbl_ovrd=off  pipeline=off  block_type=convert
  Register{,1..36,38,40..42} (41 alike) : xbsIndex_r4/Register  init=0  rst=off  en=off  dbl_ovrd=off  block_type=register
  Up Sample : xbsIndex_r4/Up Sample  sample_ratio=16  copy_samples=on  en=off  latency=0  dbl_ovrd=off  block_type=usamp
  wires:
  Register6/1 -> Register/1
  Register/1 -> ChipScope/12, ChipScope/5  "ADC_IQ_Valid"
  Register8/1 -> Register2/1
  Register9/1 -> Register3/1
  Register10/1 -> Register4/1
  Register11/1 -> Register5/1
  Register29/1 -> Register27/1
  Register38/1 -> Register36/1
  Register2/1 -> ChipScope/13  "ADC_I"
  Register3/1 -> ChipScope/14  "ADC_Q"
  Register4/1 -> ChipScope/15  "EQ_I"
  Register5/1 -> ChipScope/16  "EQ_Q"
  Register27/1 -> ChipScope/17  "RSSI"
  Register36/1 -> ChipScope/18  "OFDM_Rx_Start"
  Register23/1 -> Register40/1
  Register40/1 -> ChipScope/19  "DSSS_Rx_Start"
  Register12/1 -> Register13/1
  Register13/1 -> ChipScope/8, ChipScope/1  "FCS_Good"
  Register15/1 -> Register14/1
  Register14/1 -> ChipScope/9, ChipScope/2  "Pkt_Done"
  Register16/1 -> Register17/1
  Register17/1 -> ChipScope/10, ChipScope/3  "Pkt_Det_OFDM"
  [CS_ADC_IQ_Valid] -> Register6/1
  [CS_ADC_I] -> Convert4/1
  [CS_ADC_Q] -> Convert5/1
  [CS_EQ_I] -> Convert1/1
  [CS_EQ_Q] -> Convert2/1
  Convert1/1 -> Register10/1
  Convert2/1 -> Register11/1
  Up Sample/1 -> Register29/1
  Concat1/1 -> Register12/1
  [CS_Pktdet_OFDM] -> Register16/1
  Convert4/1 -> Register8/1
  Convert5/1 -> Register9/1
  [DSSS_FCS_GOOD] -> Concat1/1
  [OFDM_FCS_GOOD] -> Concat1/2
  [DSSS_RX_DATA_DONE] -> Concat2/1
  [OFDM_RX_DATA_DONE] -> Concat2/2
  Concat2/1 -> Register15/1
  Register1/1 -> Register7/1
  Register7/1 -> ChipScope/20  "MAC_RX_RATE"
  Register18/1 -> Register19/1
  Register19/1 -> ChipScope/21  "MAC_RX_END"
  Register20/1 -> Register21/1
  Register21/1 -> ChipScope/22  "MAC_RX_START"
  [CS_RFA_RSSI] -> Up Sample/1
  [CS_MAC_OUT_RX_RATE] -> Register1/1
  [CS_MAC_OUT_RX_START] -> Register20/1
  [CS_SW_TRIG] -> Register22/1
  Register22/1 -> ChipScope/6  "Sw Trig"
  [RX_START_OFDM_SIGNAL_VALID] -> Register38/1
  Register24/1 -> ChipScope/23  "MAC_FCS_GOOD"
  Register25/1 -> ChipScope/24  "ADC_I_RFC"
  Register26/1 -> ChipScope/25  "ADC_Q_RFC"
  [CS_MAC_OUT_FCS_GOOD] -> Register28/1
  Register28/1 -> Register24/1
  Register31/1 -> Register25/1
  Register33/1 -> Register26/1
  Register34/1 -> Register35/1
  Register35/1 -> ChipScope/26  "Phase_Err"
  [CS_DSSS_BIT_VALID] -> Register23/1
  [CS_LTS_Corr] -> Convert3/1
  [CS_Phase_Err] -> Register34/1
  [CS_ADC_Q_RFC] -> Register33/1
  [CS_ADC_I_RFC] -> Register31/1
  [CS_MAC_OUT_RX_END] -> Register18/1
  [CS_EQ_Valid] -> Register41/1
  Register41/1 -> Register42/1
  Register42/1 -> ChipScope/7  "EQ_Valid"
  Register30/1 -> Register32/1
  [CS_Pktdet_DSSS] -> Register30/1
  Register32/1 -> ChipScope/11, ChipScope/4  "Pkt_Det_DSSS"

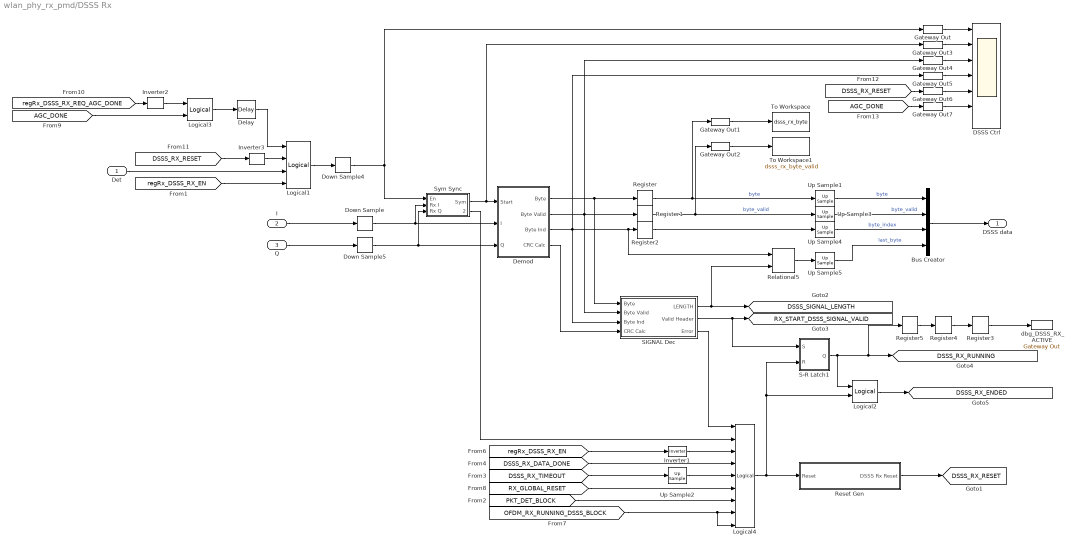
== system wlan_phy_rx_pmd/DSSS Rx ==
  Det : Inport
  I : Inport  Port=2
  Q : Inport  Port=3
  Bus Creator : BusCreator
  DSSS Ctrl : Scope  NumInputPorts=7
      settings: TimeRange=14500  YMin=0.95~-1~-1~-1~-1~0.95~-1  YMax=1.05~1~1~1~1~1.05~1  SaveName=ScopeData41
  Delay : xbsIndex_r4/Delay  rst=off  infoeditControl=Selection of Reset will increase slice count due to use of real FFs and instead of SRLs  en=off  latency=64  dbl_ovrd=off  reg_retiming=off  block_type=delay
  Demod : Subsystem
  Down Sample : xbsIndex_r4/Down Sample  sample_ratio=8  sample_phase="Last Value of Frame  (most efficient)"  en=off  latency=1  dbl_ovrd=off  block_type=dsamp
  Down Sample4 : xbsIndex_r4/Down Sample  sample_ratio=8  sample_phase="Last Value of Frame  (most efficient)"  en=off  latency=1  dbl_ovrd=off  block_type=dsamp
  Down Sample5 : xbsIndex_r4/Down Sample  sample_ratio=8  sample_phase="Last Value of Frame  (most efficient)"  en=off  latency=1  dbl_ovrd=off  block_type=dsamp
  Gateway Out{,1..7} (8 alike) : xbsIndex_r4/Gateway Out  inherit_from_input=off  hdl_port=off  timing_constraint=None  locs_specified=off  LOCs={}  UseAsDAC=off  DACChannel='1'  block_type=gatewayout
  Inverter1 : xbsIndex_r4/Inverter  en=off  latency=0  dbl_ovrd=off  block_type=inv
  Inverter2 : xbsIndex_r4/Inverter  en=off  latency=0  dbl_ovrd=off  block_type=inv
      settings: xl_area=[0 0 0 0 0 0 0]  block_version=VER_STRING_GOES_HERE
  Inverter3 : xbsIndex_r4/Inverter  en=off  latency=0  dbl_ovrd=off  block_type=inv
      settings: xl_area=[0 0 0 0 0 0 0]  block_version=VER_STRING_GOES_HERE
  Logical1 : xbsIndex_r4/Logical  logical_function=AND  inputs=4  en=off  latency=0  precision=Full  arith_type=Unsigned  n_bits=16  bin_pt=0  align_bp=on  dbl_ovrd=off  block_type=logical
  Logical2 : xbsIndex_r4/Logical  logical_function=AND  inputs=2  en=off  latency=0  precision=Full  arith_type=Unsigned  n_bits=16  bin_pt=0  align_bp=on  dbl_ovrd=off  block_type=logical
  Logical3 : xbsIndex_r4/Logical  logical_function=OR  inputs=2  en=off  latency=0  precision=Full  arith_type=Unsigned  n_bits=16  bin_pt=0  align_bp=on  dbl_ovrd=off  block_type=logical
  Logical4 : xbsIndex_r4/Logical  logical_function=OR  inputs=9  en=off  latency=0  precision=Full  arith_type=Unsigned  n_bits=16  bin_pt=0  align_bp=on  dbl_ovrd=off  block_type=logical
  Register{,1..5} (6 alike) : xbsIndex_r4/Register  init=0  rst=off  en=off  dbl_ovrd=off  block_type=register
  Relational5 : xbsIndex_r4/Relational  mode=a>b  en=off  latency=0  dbl_ovrd=off  block_type=relational
  Reset Gen : Subsystem
  S-R Latch1 : Subsystem
  SIGNAL Dec : Subsystem
  Sym Sync : Subsystem
  To Workspace : ToWorkspace  VariableName=dsss_rx_byte  SampleTime=-1  SaveFormat=Array
  To Workspace1 : ToWorkspace  VariableName=dsss_rx_byte_valid  SampleTime=-1  SaveFormat=Array
  Up Sample{1..5} (5 alike) : xbsIndex_r4/Up Sample  sample_ratio=8  copy_samples=on  en=off  latency=0  dbl_ovrd=off  block_type=usamp
  dbg_DSSS_RX_ACTIVE : xbsIndex_r4/Gateway Out  inherit_from_input=off  hdl_port=on  timing_constraint=None  locs_specified=off  LOCs={}  UseAsDAC=off  DACChannel='1'  block_type=gatewayout
  DSSS data : Outport
  tag scope: DSSS_RX_ENDED=global, DSSS_RX_RESET=global, DSSS_RX_RUNNING=global, DSSS_SIGNAL_LENGTH=global, RX_START_DSSS_SIGNAL_VALID=global
  wires:
  Sym Sync/1(Sym) -> Demod/1(Start), Gateway Out3/1
  I/1 -> Down Sample/1
  Q/1 -> Down Sample5/1
  Demod/1(Byte) -> Register/1, SIGNAL Dec/1(Byte)
  Demod/2(Byte Valid) -> Register1/1, SIGNAL Dec/2(Byte Valid), Gateway Out4/1
  Demod/3(Byte Ind) -> Relational5/1, Register2/1, SIGNAL Dec/3(Byte Ind), Gateway Out5/1
  Demod/4(CRC Calc) -> SIGNAL Dec/4(CRC Calc)
  Relational5/1 -> Up Sample5/1
  Register/1 -> Gateway Out1/1, Up Sample1/1  "byte"
  Register1/1 -> Gateway Out2/1, Up Sample3/1  "byte_valid"
  Register2/1 -> Up Sample4/1
  SIGNAL Dec/1(LENGTH) -> Relational5/2, [DSSS_SIGNAL_LENGTH]
  Gateway Out1/1 -> To Workspace/1
  Gateway Out2/1 -> To Workspace1/1
  Down Sample/1 -> Demod/2(I), Sym Sync/2(Rx I)
  Down Sample5/1 -> Demod/3(Q), Sym Sync/3(Rx Q)
  Logical1/1 -> Down Sample4/1
  Down Sample4/1 -> Sym Sync/1(En), Gateway Out/1
  Bus Creator/1 -> DSSS data/1
  [DSSS_RX_DATA_DONE] -> Logical4/4
  [DSSS_RX_TIMEOUT] -> Up Sample2/1
  Logical4/1 -> Reset Gen/1(Reset), S-R Latch1/2(R), Logical2/2
  [regRx_DSSS_RX_EN] -> Inverter1/1
  [RX_GLOBAL_RESET] -> Logical4/6
  SIGNAL Dec/2(Valid Header) -> [RX_START_DSSS_SIGNAL_VALID], S-R Latch1/1(S)
  Inverter1/1 -> Logical4/3
  SIGNAL Dec/3(Error) -> Logical4/1
  Up Sample2/1 -> Logical4/5
  [PKT_DET_BLOCK] -> Logical4/7
  Up Sample1/1 -> Bus Creator/1  "byte"
  Up Sample3/1 -> Bus Creator/2  "byte_valid"
  Up Sample4/1 -> Bus Creator/3  "byte_index"
  Up Sample5/1 -> Bus Creator/4  "last_byte"
  S-R Latch1/1(Q) -> Logical2/1, [DSSS_RX_RUNNING], Register5/1
  [OFDM_RX_RUNNING_DSSS_BLOCK] -> Logical4/9, Logical4/8
  Reset Gen/1(DSSS Rx Reset) -> [DSSS_RX_RESET]
  Logical2/1 -> [DSSS_RX_ENDED]
  Register3/1 -> dbg_DSSS_RX_ACTIVE/1
  Register4/1 -> Register3/1
  Register5/1 -> Register4/1
  [regRx_DSSS_RX_EN] -> Logical1/4
  [AGC_DONE] -> Logical3/2
  [regRx_DSSS_RX_REQ_AGC_DONE] -> Inverter2/1
  Logical3/1 -> Delay/1
  [DSSS_RX_RESET] -> Inverter3/1
  Inverter3/1 -> Logical1/2
  Gateway Out/1 -> DSSS Ctrl/1
  Gateway Out3/1 -> DSSS Ctrl/2
  Gateway Out4/1 -> DSSS Ctrl/3
  Gateway Out5/1 -> DSSS Ctrl/4
  [DSSS_RX_RESET] -> Gateway Out6/1
  Gateway Out6/1 -> DSSS Ctrl/5
  [AGC_DONE] -> Gateway Out7/1
  Gateway Out7/1 -> DSSS Ctrl/6
  Det/1 -> Logical1/3
  Inverter2/1 -> Logical3/1
  Delay/1 -> Logical1/1
  Sym Sync/2(Timeout) -> Logical4/2

== system wlan_phy_rx_pmd/Detect & Decode ==
  note: Flush the OFDM pipeline if DSSS Rx starts
  Eq I : Inport
  Eq Q : Inport  Port=2
  x_ind : Inport  Port=3
  x_valid : Inport  Port=4
  ofdm_sym_ind : Inport  Port=5
  Bus Creator : BusCreator
  Ctrl : Subsystem
  De-Interleave & De-Puncture : Subsystem
  Decode : Subsystem
  Descramble : Subsystem
  Logical1 : xbsIndex_r4/Logical  logical_function=OR  inputs=2  en=off  latency=0  precision=Full  arith_type=Unsigned  n_bits=16  bin_pt=0  align_bp=on  dbl_ovrd=off  block_type=logical
  Logical5 : xbsIndex_r4/Logical  logical_function=OR  inputs=2  en=off  latency=0  precision=Full  arith_type=Unsigned  n_bits=16  bin_pt=0  align_bp=on  dbl_ovrd=off  block_type=logical
  Mod Rate Sel : Subsystem
  Soft Demap : Subsystem
  Subcarrier Map : Subsystem
  Sym FIFO : Subsystem
  OFDM Data : Outport
  " Reset" : Outport  Port=2
  wires:
  Eq I/1 -> Sym FIFO/4(I)
  Eq Q/1 -> Sym FIFO/5(Q)
  x_ind/1 -> Subcarrier Map/2(x_ind)
  x_valid/1 -> Subcarrier Map/1(x_valid)
  [RX_GLOBAL_RESET] -> Logical5/2
  Logical5/1 -> Decode/1(Reset), Ctrl/1(Reset), Sym FIFO/2(Reset)
  Subcarrier Map/1(data_sym_valid) -> Sym FIFO/3(tvalid)
  Sym FIFO/1(" tvalid") -> Soft Demap/1("tvalid ")
  Sym FIFO/2(" I") -> Soft Demap/2(I)
  Sym FIFO/3(" Q") -> Soft Demap/3(Q)
  Mod Rate Sel/1(Mod Sel) -> Soft Demap/5(mod_sel)
  Soft Demap/1(tready) -> Sym FIFO/1(tready)
  Soft Demap/2(LLR A) -> De-Interleave & De-Puncture/1(LLR A)
  Soft Demap/4(tvalid) -> De-Interleave & De-Puncture/3(tvalid)
  Soft Demap/5(" mod_sel") -> De-Interleave & De-Puncture/4(mod_sel)
  De-Interleave & De-Puncture/1(" LLR A") -> Decode/2(LLR A)
  De-Interleave & De-Puncture/2(" LLR B") -> Decode/3(LLR B)
  De-Interleave & De-Puncture/3(" tvalid") -> Decode/4(Vin)
  Ctrl/2(Start Decoder) -> Decode/5(Start)
  Decode/2(Byte Valid) -> Descramble/2(Byte Valid)
  ofdm_sym_ind/1 -> Mod Rate Sel/1(OFDM Sym Ind), Ctrl/2(OFDM Sym Ind), Sym FIFO/6(OFDM Sym)
  Sym FIFO/4(Full Rate) -> Soft Demap/4(Full Rate)
  Ctrl/1(Reset Demod) -> De-Interleave & De-Puncture/5(Reset), Soft Demap/6(Reest)
  Decode/3(Byte Index) -> Descramble/3(Byte Index)
  Decode/4(Last Byte) -> Descramble/4(Last Byte)
  Decode/1(Byte) -> Descramble/1(Byte)
  Decode/6(Mod Order) -> Mod Rate Sel/2(Payload Mod)
  Decode/7(Code Rate) -> De-Interleave & De-Puncture/6(rate_sel)
  Decode/5(" Reset") -> Logical1/1
  Bus Creator/1 -> OFDM Data/1
  Descramble/1(" Byte") -> Bus Creator/1  "byte"
  Descramble/2(" Byte Valid") -> Bus Creator/2  "byte_valid"
  Descramble/3(" Byte Index") -> Bus Creator/3  "byte_index"
  Descramble/4(" Last Byte") -> Bus Creator/4  "last_byte"
  Soft Demap/3(LLR B) -> De-Interleave & De-Puncture/2(LLR B)
  [DSSS_RX_RUNNING] -> Logical1/2
  Logical1/1 -> " Reset"/1, Logical5/1
